simulink model "cddDcmApp"  (R2022b)
settings: Solver=FixedStepDiscrete  StartTime=0.0  StopTime=10.0  FixedStep=0.02  RelTol=1e-3
undefined symbols (not defined in the file or in its folder): CCPFaultType, DcChlType, HFAChlType, IcuChannelType, MtrRoutStaEnum, P_SignalPartType, P_Std_ReturnType, PowerChlType, SrsAdChlType, VGAChlType
(part 1 of 13)
settings Model: Creator=blue.ge  LastModifiedBy=blue.ge
settings DataIOCC: SaveFormat=StructureWithTime
settings OptimizationCC: DefaultParameterBehavior=Inlined  EnhancedBackFolding=on  PassReuseOutputArgsAs=Individual arguments  ZeroExternalMemoryAtStartup=off  ZeroInternalMemoryAtStartup=off  OptimizeBlockOrder=speed  DifferentSizesBufferReuse=on  OptimizationCustomize=off  EfficientTunableParamExpr=on
settings DebuggingCC: ParameterTunabilityLossMsg=error
settings ModelReferenceCC: ModelReferenceNumInstancesAllowed=Single
settings SFSimCC: MATLABDynamicMemAlloc=off
settings RTWCC: Description=AUTOSAR  SystemTargetFile=autosar.tlc  GenCodeOnly=on  TemplateMakefile=ert_default_tmf
settings CodeAppCC: ForceParamTrailComments=on  IgnoreCustomStorageClasses=off  OperatorAnnotations=on  SimulinkDataObjDesc=on  SFDataObjDesc=on  InsertBlockDesc=on
settings STFCustomTargetCC: TargetLangStandard=C99 (ISO)  MultiwordLength=256  ParMdlRefBuildCompliant=on  CompOptLevelCompliant=on  IncludeMdlTerminateFcn=off  CombineOutputUpdateFcns=on  SuppressErrorStatus=on  ERTCustomFileBanners=on  SupportNonFinite=off  SupportComplex=off  SupportContinuousTime=off  SupportNonInlinedSFcns=off  RemoveResetFunc=on  MATLABClassNameForMDSCustomization=Simulink.SoftwareTarget.ERTCustomization  AutosarCompliant=on  UseToolchainInfoCompliant=on  LookupTableObjectStructAxisOrder=2,1,3,4,...  LUTObjectStructOrderEvenSpacing=Size,Table,Breakpoints  SystemTargetFile=autosar.tlc
settings ERTTargetCC: TargetLangStandard=C99 (ISO)  ConcurrentExecutionCompliant=off  IncludeMdlTerminateFcn=off  SuppressErrorStatus=on  SupportNonFinite=off  SupportComplex=off  ModelStepFunctionPrototypeControlCompliant=off  CPPClassGenCompliant=off  AutosarCompliant=on  LookupTableObjectStructAxisOrder=2,1,3,4,...  LUTObjectStructOrderEvenSpacing=Size,Table,Breakpoints  InlinedParameterPlacement=NonHierarchical  ERTCustomFileTemplate=example_file_process.tlc

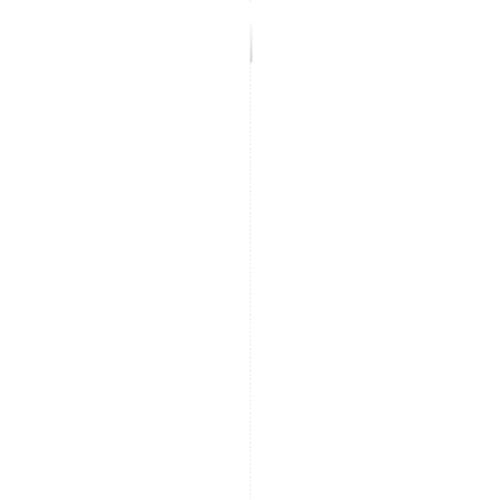
[thumbnail: Simulink's stored preview of the top-level diagram]
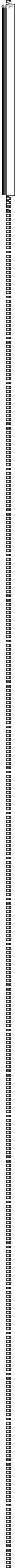
== system cddDcmApp ==
  cddDcmApp_20ms_Runnable : Inport  SampleTime=0.02  OutputFunctionCall=on
  cddDcmApp_Init : Inport  Port=2  OutputFunctionCall=on
  Dcm_Reset_Hard : Inport  Port=3  OutputFunctionCall=on
  Dcm_Reset_JumpToBootLoader : Inport  Port=4  OutputFunctionCall=on
  DiagSessionCtrl_Default : Inport  Port=5  OutputFunctionCall=on
  AACAirClngErrStsNgcc_AACAirClngErrStsNgcc : Inport  Port=6  OutDataTypeStr=Enum: AACAirClngErrSts  PortDimensions=1
  AACAirClngStsNgcc_AACAirClngStsNgcc : Inport  Port=7  OutDataTypeStr=Enum: AACAirClngSts  PortDimensions=1
  AfuFrameSts_AfuFrameSts : Inport  Port=8  OutDataTypeStr=UInt8  PortDimensions=1
  AFUGenErr_AFUGenErr : Inport  Port=9  OutDataTypeStr=Enum: OnOff1  PortDimensions=1
  AirClngErrSts_AirClngErrSts : Inport  Port=10  OutDataTypeStr=Enum: AirClngErrSts  PortDimensions=1
  AirClngSts_AirClngSts : Inport  Port=11  OutDataTypeStr=Enum: AirIonSts  PortDimensions=1
  AirFragFanErr_AirFragFanErr : Inport  Port=12  OutDataTypeStr=Enum: Err1  PortDimensions=1
  AirFragMot1Err_AirFragMot1Err : Inport  Port=13  OutDataTypeStr=Enum: Err1  PortDimensions=1
  AirFragMot2Err_AirFragMot2Err : Inport  Port=14  OutDataTypeStr=Enum: Err1  PortDimensions=1
  AirFragMot3Err_AirFragMot3Err : Inport  Port=15  OutDataTypeStr=Enum: Err1  PortDimensions=1
  AirFragMot4Err_AirFragMot4Err : Inport  Port=16  OutDataTypeStr=Enum: Err1  PortDimensions=1
  AirFragMot5Err_AirFragMot5Err : Inport  Port=17  OutDataTypeStr=Enum: Err1  PortDimensions=1
  AirFragOvrVoltErr_AirFragOvrVoltErr : Inport  Port=18  OutDataTypeStr=Enum: Err1  PortDimensions=1
  AirFragTempErr_AirFragTempErr : Inport  Port=19  OutDataTypeStr=Enum: TempErr  PortDimensions=1
  AirFragUndVoltErr_AirFragUndVoltErr : Inport  Port=20  OutDataTypeStr=Enum: Err1  PortDimensions=1
  AirTFromHeatgEstimdFirstLeForCCM_AirTFromHeatgEstimdFirstLeForCCM : Inport  Port=21  OutDataTypeStr=T30  PortDimensions=1
  AirTFromHeatgEstimdFirstRiForCCM_AirTFromHeatgEstimdFirstRiForCCM : Inport  Port=22  OutDataTypeStr=T30  PortDimensions=1
  AirTFromHeatgEstimdForCCMForRead_AirTFromHeatgEstimdForCCMForRead : Inport  Port=23  OutDataTypeStr=T30  PortDimensions=1
  AirTFromHeatgEstimdReForCCM_AirTFromHeatgEstimdReForCCM : Inport  Port=24  OutDataTypeStr=T30  PortDimensions=1
  AirTFromHeatgEstimdSecLeForCCM_AirTFromHeatgEstimdSecLeForCCM : Inport  Port=25  OutDataTypeStr=T30  PortDimensions=1
  AiuFrameSts_AiuFrameSts : Inport  Port=26  OutDataTypeStr=UInt8  PortDimensions=1
  AmbTFildForClimaNgcc_AmbTFildForClima : Inport  Port=27  OutDataTypeStr=T30  PortDimensions=1
  AmbTRaw_AmbTRaw : Inport  Port=28  OutDataTypeStr=Bus: AmbTWithQly  PortDimensions=1  BusOutputAsStruct=on
  AQSAirQuality_AQSAirQuality : Inport  Port=29  OutDataTypeStr=Enum: AQSAirQuality  PortDimensions=1
  AQSCOxstatus_AQSCOxstatus : Inport  Port=30  OutDataTypeStr=Enum: AQSAirQuality  PortDimensions=1
  AqsFrameSts_AqsFrameSts : Inport  Port=31  OutDataTypeStr=UInt8  PortDimensions=1
  AQSNH3status_AQSNH3status : Inport  Port=32  OutDataTypeStr=Enum: AQSAirQuality  PortDimensions=1
  AQSNoxStatus_AQSNoxStatus : Inport  Port=33  OutDataTypeStr=Enum: AQSAirQuality  PortDimensions=1
  BkpOfDstTrvld_BkpOfDstTrvld : Inport  Port=34  OutDataTypeStr=Len31  PortDimensions=1
  BkpOfDstTrvld_BkpOfDstTrvld_IsUpdated : Inport  Port=35  OutDataTypeStr=boolean  PortDimensions=1
  CarCfgForClimaCtrl_CarCfgForAirClng : Inport  Port=36  OutDataTypeStr=Enum: CarCfgForAirClng  PortDimensions=1
  CarCfgForClimaCtrl_CarCfgForAirClnrAdv : Inport  Port=37  OutDataTypeStr=UInt8  PortDimensions=1
  CarCfgForClimaCtrl_CarCfgForAirFrag : Inport  Port=38  OutDataTypeStr=Enum: CarCfgForAirFrag  PortDimensions=1
  CarCfgForClimaCtrl_CarCfgForAirMtrlSnsr : Inport  Port=39  OutDataTypeStr=Enum: CarCfgForAirMtrlSnsrGly  PortDimensions=1
  CarCfgForClimaCtrl_CarCfgForAirQly : Inport  Port=40  OutDataTypeStr=Enum: CarCfgForAirQlyGly  PortDimensions=1
  CarCfgForClimaCtrl_CarCfgForAmntOfSeat : Inport  Port=41  OutDataTypeStr=Enum: CarCfgForAmntOfSeatTyp3  PortDimensions=1
  CarCfgForClimaCtrl_CarCfgForAutDemst : Inport  Port=42  OutDataTypeStr=UInt8  PortDimensions=1
  CarCfgForClimaCtrl_CarCfgForAuxWtrPmp : Inport  Port=43  OutDataTypeStr=Enum: CarCfgForAuxWtrPmp  PortDimensions=1
  CarCfgForClimaCtrl_CarCfgForBRAND : Inport  Port=44  OutDataTypeStr=UInt8  PortDimensions=1
  CarCfgForClimaCtrl_CarCfgForBypVlvInHvac : Inport  Port=45  OutDataTypeStr=Enum: CarCfgForBypVlvInHvac2  PortDimensions=1
  CarCfgForClimaCtrl_CarCfgForCabinOxgenSupply : Inport  Port=46  OutDataTypeStr=UInt8  PortDimensions=1
  CarCfgForClimaCtrl_CarCfgForClsVlvInHvac : Inport  Port=47  OutDataTypeStr=Enum: CarCfgForClsVlvInHvac  PortDimensions=1
  CarCfgForClimaCtrl_CarCfgForCmprAcTyp : Inport  Port=48  OutDataTypeStr=UInt8  PortDimensions=1
  CarCfgForClimaCtrl_CarCfgForCmptmtClng : Inport  Port=49  OutDataTypeStr=Enum: CarCfgForCmptmtClng  PortDimensions=1
  CarCfgForClimaCtrl_CarCfgForElectricLiftingModule : Inport  Port=50  OutDataTypeStr=UInt8  PortDimensions=1
  CarCfgForClimaCtrl_CarCfgForElecVentAdjmt : Inport  Port=51  OutDataTypeStr=Enum: CarCfgForElecVentAdjmt  PortDimensions=1
  CarCfgForClimaCtrl_CarCfgForExtVentnClimaCtrl : Inport  Port=52  OutDataTypeStr=Enum: CarCfgForExtVentnClimaCtrl  PortDimensions=1
  CarCfgForClimaCtrl_CarCfgForFlcTyp : Inport  Port=53  OutDataTypeStr=Enum: CarCfgForFlcTyp  PortDimensions=1
  CarCfgForClimaCtrl_CarCfgForFloatingDriving : Inport  Port=54  OutDataTypeStr=UInt8  PortDimensions=1
  CarCfgForClimaCtrl_CarCfgForFoldCSD : Inport  Port=55  OutDataTypeStr=Enum: CarCfgForFoldCSD  PortDimensions=1
  CarCfgForClimaCtrl_CarCfgForFuTyp : Inport  Port=56  OutDataTypeStr=Enum: CarCfgForFuTyp  PortDimensions=1
  CarCfgForClimaCtrl_CarCfgForGlassTyp : Inport  Port=57  OutDataTypeStr=Enum: CarCfgForGlassTyp  PortDimensions=1
  CarCfgForClimaCtrl_CarCfgForHeadRadar : Inport  Port=58  OutDataTypeStr=Enum: HeadRadar  PortDimensions=1
  CarCfgForClimaCtrl_CarCfgForHeatPmp : Inport  Port=59  OutDataTypeStr=Enum: CarCfgForHeatPmpTyp  PortDimensions=1
  CarCfgForClimaCtrl_CarCfgForHeatrDurgDrvg : Inport  Port=60  OutDataTypeStr=Enum: CarCfgForHeatrDurgDrvg  PortDimensions=1
  CarCfgForClimaCtrl_CarCfgForHeatrTyp : Inport  Port=61  OutDataTypeStr=UInt8  PortDimensions=1
  CarCfgForClimaCtrl_CarCfgForHvacFilTyp : Inport  Port=62  OutDataTypeStr=Enum: CarCfgForHvacFilTypGly  PortDimensions=1
  CarCfgForClimaCtrl_CarCfgForHvacFlrSnsrForT : Inport  Port=63  OutDataTypeStr=Enum: CarCfgForHvacFlrSnsrForT  PortDimensions=1
  CarCfgForClimaCtrl_CarCfgForHvacHeatrSnsrT : Inport  Port=64  OutDataTypeStr=Enum: CarCfgForHvacHeatrSnsrT  PortDimensions=1
  CarCfgForClimaCtrl_CarCfgForHvacRe : Inport  Port=65  OutDataTypeStr=UInt8  PortDimensions=1
  CarCfgForClimaCtrl_CarCfgForHvacTyp : Inport  Port=66  OutDataTypeStr=UInt8  PortDimensions=1
  CarCfgForClimaCtrl_CarCfgForInteriorViewCamera : Inport  Port=67  OutDataTypeStr=Enum: CarCfgForInteriorViewCamera  PortDimensions=1
  CarCfgForClimaCtrl_CarCfgForLifeDetection : Inport  Port=68  OutDataTypeStr=Enum: LifeDetection  PortDimensions=1
  CarCfgForClimaCtrl_CarCfgForLoVoltElecCfg : Inport  Port=69  OutDataTypeStr=Enum: CarCfgForLoVoltElecCfg  PortDimensions=1
  CarCfgForClimaCtrl_CarCfgForNavSysandIntnmap : Inport  Port=70  OutDataTypeStr=Enum: CarCfgForNavSysandIntnmap  PortDimensions=1
  CarCfgForClimaCtrl_CarCfgForNrOfDoor : Inport  Port=71  OutDataTypeStr=Enum: CarCfgForNrOfDoor2  PortDimensions=1
  CarCfgForClimaCtrl_CarCfgForPipeInFuTank : Inport  Port=72  OutDataTypeStr=Enum: CarCfgForPipeInFuTank  PortDimensions=1
  CarCfgForClimaCtrl_CarCfgForPowerSeats : Inport  Port=73  OutDataTypeStr=Enum: CarCfgForPowerSeats  PortDimensions=1
  CarCfgForClimaCtrl_CarCfgForPreClng : Inport  Port=74  OutDataTypeStr=Enum: CarCfgForPreClng  PortDimensions=1
  CarCfgForClimaCtrl_CarCfgForPrkgCDSNotAvl : Inport  Port=75  OutDataTypeStr=Enum: CarCfgForPrkgCDSNotAvl  PortDimensions=1
  CarCfgForClimaCtrl_CarCfgForPrkgClima : Inport  Port=76  OutDataTypeStr=Enum: CarCfgForPrkgClima  PortDimensions=1
  CarCfgForClimaCtrl_CarCfgForPrpsnTyp : Inport  Port=77  OutDataTypeStr=Enum: CarCfgForPrpsnTypGly  PortDimensions=1
  CarCfgForClimaCtrl_CarCfgForPTCInFrontHVAC : Inport  Port=78  OutDataTypeStr=UInt8  PortDimensions=1
  CarCfgForClimaCtrl_CarCfgForRemEngStrtPreCdn : Inport  Port=79  OutDataTypeStr=Enum: CarCfgForRemEngStrtPreCdn  PortDimensions=1
  CarCfgForClimaCtrl_CarCfgForRoofHatch : Inport  Port=80  OutDataTypeStr=Enum: CarCfgForRoofHatch  PortDimensions=1
  CarCfgForClimaCtrl_CarCfgForSeatHeatgCal : Inport  Port=81  OutDataTypeStr=Enum: CarCfgForSeatHeatgCal1  PortDimensions=1
  CarCfgForClimaCtrl_CarCfgForSeatHeatgForRowFirst : Inport  Port=82  OutDataTypeStr=Enum: CarCfgForSeatHeatgForRowFirst  PortDimensions=1
  CarCfgForClimaCtrl_CarCfgForSeatHeatgForRowSec : Inport  Port=83  OutDataTypeStr=Enum: CarCfgForSeatHeatgForRowSec  PortDimensions=1
  CarCfgForClimaCtrl_CarCfgForSeatVentnForRowFirst : Inport  Port=84  OutDataTypeStr=Enum: CarCfgForSeatVentnForRowFirst1  PortDimensions=1
  CarCfgForClimaCtrl_CarCfgForSeatVentnForRowSec : Inport  Port=85  OutDataTypeStr=Enum: CarCfgForSeatVentnForRowSecGly  PortDimensions=1
  CarCfgForClimaCtrl_CarCfgForSecRowVentnCenter : Inport  Port=86  OutDataTypeStr=Enum: CarCfgForSecRowVentnCenter  PortDimensions=1
  CarCfgForClimaCtrl_CarCfgForSecRowVentnSide : Inport  Port=87  OutDataTypeStr=Enum: CarCfgForSecRowVentnSide  PortDimensions=1
  CarCfgForClimaCtrl_CarCfgForSmallFlowCyc : Inport  Port=88  OutDataTypeStr=Enum: CarCfgForSmallFlowCyc  PortDimensions=1
  CarCfgForClimaCtrl_CarCfgForSmkSnsr : Inport  Port=89  OutDataTypeStr=Enum: CarCfgForSmkSnsr  PortDimensions=1
  CarCfgForClimaCtrl_CarCfgForSnsrForCmptmtT : Inport  Port=90  OutDataTypeStr=Enum: CarCfgForSnsrForCmptmtT  PortDimensions=1
  CarCfgForClimaCtrl_CarCfgForSnsrHumPrsnt : Inport  Port=91  OutDataTypeStr=Enum: CarCfgForSnsrHumPrsnt  PortDimensions=1
  CarCfgForClimaCtrl_CarCfgForStartDevice : Inport  Port=92  OutDataTypeStr=Enum: StartDevice  PortDimensions=1
  CarCfgForClimaCtrl_CarCfgForSteerWhlHeatg : Inport  Port=93  OutDataTypeStr=UInt8  PortDimensions=1
  CarCfgForClimaCtrl_CarCfgForSteerWhlPosn : Inport  Port=94  OutDataTypeStr=Enum: CarCfgForSteerWhlPosn  PortDimensions=1
  CarCfgForClimaCtrl_CarCfgForSunSnsr : Inport  Port=95  OutDataTypeStr=Enum: CarCfgForSunSnsr  PortDimensions=1
  CarCfgForClimaCtrl_CarCfgForTelmNodTyp : Inport  Port=96  OutDataTypeStr=Enum: CarCfgForTelmNodTyp  PortDimensions=1
  CarCfgForClimaCtrl_CarCfgForTEMPEREDSTORAGEBOX : Inport  Port=97  OutDataTypeStr=UInt8  PortDimensions=1
  CarCfgForClimaCtrl_CarCfgForThermMngt : Inport  Port=98  OutDataTypeStr=Enum: CarCfgForThermMngt  PortDimensions=1
  CarCfgForClimaCtrl_CarCfgForVehTyp : Inport  Port=99  OutDataTypeStr=Enum: CarCfgForVehTyp3  PortDimensions=1
  CarCfgForClimaCtrl_CarCfgForVentLiftMotorExiSts : Inport  Port=100  OutDataTypeStr=UInt32  PortDimensions=1
  CarCfgForClimaCtrl_CarCfgForVgaMotorExistSts : Inport  Port=101  OutDataTypeStr=CarCfgForVgaMotorExistSts  PortDimensions=1
  CarCfgForClimaCtrl_CarCfgForWadingAssistSystem : Inport  Port=102  OutDataTypeStr=UInt8  PortDimensions=1
  CarCfgForClimaCtrl_CarCfgForWindHeatd : Inport  Port=103  OutDataTypeStr=Enum: CarCfgForWindHeatd  PortDimensions=1
  CarCfgForClimaCtrl_CarCfgWindFollowHumanMov : Inport  Port=104  OutDataTypeStr=UInt8  PortDimensions=1
  CarTiGlb_CarTiGlb : Inport  Port=105  OutDataTypeStr=CarTiGlb  PortDimensions=1
  CarTiGlb_CarTiGlb_IsUpdated : Inport  Port=106  OutDataTypeStr=boolean  PortDimensions=1
  CcmIntCo2Lvl_CcmIntCo2Lvl : Inport  Port=107  OutDataTypeStr=Enum: Co2Lvl  PortDimensions=1
  CCMLIN1frameSts_CCMLIN1frameSts : Inport  Port=108  OutDataTypeStr=UInt8  PortDimensions=8
  CCMLIN2frameSts_CCMLIN2frameSts : Inport  Port=109  OutDataTypeStr=UInt8  PortDimensions=8
  CCMLIN3frameSts_CCMLIN3frameSts : Inport  Port=110  OutDataTypeStr=UInt8  PortDimensions=8
  CdsFrameSts_CdsFrameSts : Inport  Port=111  OutDataTypeStr=UInt8  PortDimensions=1
  ClimaObsActv_ClimaObsActv : Inport  Port=112  OutDataTypeStr=Boolean  PortDimensions=1
  CmpmtInAirPmAQI_CmpmtInAirPmAQI : Inport  Port=113  OutDataTypeStr=Enum: CmpmtAirPmLvl  PortDimensions=1
  CmpmtInAirPmDnsty_CmpmtInAirPmDnsty : Inport  Port=114  OutDataTypeStr=UInt10  PortDimensions=1
  CmptmtAirTEstimdAtRowFirst_CmptmtAirTEstimdAtRowFirst : Inport  Port=115  OutDataTypeStr=T30  PortDimensions=1
  CmptmtAirTEstimdAtRowFirstIni_CmptmtAirTEstimdAtRowFirstIni : Inport  Port=116  OutDataTypeStr=SInt8  PortDimensions=1
  CmptmtAirTEstimdAtRowSec_CmptmtAirTEstimdAtRowSec : Inport  Port=117  OutDataTypeStr=T30  PortDimensions=1
  CmptmtAirTEstimdAtRowSecIni_CmptmtAirTEstimdAtRowSecIni : Inport  Port=118  OutDataTypeStr=SInt8  PortDimensions=1
  CmptmtAirTEstimdFirstNvRamIni_CmptmtAirTEstimdFirstNvRamIni : Inport  Port=119  OutDataTypeStr=SInt8  PortDimensions=1
  CmptmtAirTEstimdSecNvRamIni_CmptmtAirTEstimdSecNvRamIni : Inport  Port=120  OutDataTypeStr=SInt8  PortDimensions=1
  CmptmtFrntWindDewT_CmptmtFrntWindDewT : Inport  Port=121  OutDataTypeStr=TByCmptmtSnsrRelHum  PortDimensions=1
  CmptmtFrntWindT_CmptmtFrntWindT : Inport  Port=122  OutDataTypeStr=TByCmptmtSnsrRelHum  PortDimensions=1
  CmptmtOxygenConcVluNgcc_CmptmtOxygenConcVluNgcc : Inport  Port=123  OutMin=0  OutMax=100  OutDataTypeStr=Perc9  PortDimensions=1
  CmptmtRelHum_CmptmtRelHum : Inport  Port=124  OutMin=0  OutMax=100  OutDataTypeStr=Perc2  PortDimensions=1
  CmptmtRelHum_CmptmtRelHum_IsUpdated : Inport  Port=125  OutDataTypeStr=boolean  PortDimensions=1
  CmptmtTRearRaw_CmptmtTRearRaw : Inport  Port=126  OutDataTypeStr=Bus: CmptmtTFrnt  PortDimensions=1  BusOutputAsStruct=on
  CoupldCtrlErrAtRowFirstLe_CoupldCtrlErrAtRowFirstLe : Inport  Port=127  OutMin=-256  OutMax=255.9  OutDataTypeStr=T2  PortDimensions=1
  CoupldCtrlErrAtRowFirstRi_CoupldCtrlErrAtRowFirstRi : Inport  Port=128  OutMin=-256  OutMax=255.9  OutDataTypeStr=T2  PortDimensions=1
  CoupldCtrlErrAtRowSecLe_CoupldCtrlErrAtRowSecLe : Inport  Port=129  OutMin=-256  OutMax=255.9  OutDataTypeStr=T2  PortDimensions=1
  CoupldCtrlErrAtRowSecRi_CoupldCtrlErrAtRowSecRi : Inport  Port=130  OutMin=-256  OutMax=255.9  OutDataTypeStr=T2  PortDimensions=1
  DcMotorCurPosVol_DcMotorCurPosVol : Inport  Port=131  OutDataTypeStr=UInt16  PortDimensions=7
  EngT_Ngcc_EngT : Inport  Port=132  OutDataTypeStr=Bus: EngT  PortDimensions=1  BusOutputAsStruct=on
  EvaprTFrntFildForClima_EvaprTFrntFildForClima : Inport  Port=133  OutDataTypeStr=T30  PortDimensions=1
  EvaprTReFildForClima_EvaprTReFildForClima : Inport  Port=134  OutDataTypeStr=T30  PortDimensions=1
  HmiHvacFanLvlFrnt_HmiHvacFanLvlFrnt : Inport  Port=135  OutDataTypeStr=Enum: HmiHvacFanLvl  PortDimensions=1
  HmiHvacFanLvlRe_HmiHvacFanLvlRe : Inport  Port=136  OutDataTypeStr=Enum: HmiHvacFanLvl  PortDimensions=1
  HvacAirTEstimdAtRowFirstLe_HvacAirTEstimdAtRowFirstLe : Inport  Port=137  OutDataTypeStr=T30  PortDimensions=1
  HvacAirTEstimdAtRowFirstRi_HvacAirTEstimdAtRowFirstRi : Inport  Port=138  OutDataTypeStr=T30  PortDimensions=1
  HvacAirTEstimdAtRowSecLe_HvacAirTEstimdAtRowSecLe : Inport  Port=139  OutDataTypeStr=T30  PortDimensions=1
  HvacAirTEstimdAtRowSecRi_HvacAirTEstimdAtRowSecRi : Inport  Port=140  OutDataTypeStr=T30  PortDimensions=1
  HvacAirTReqAtRowFirstLe_HvacAirTReqAtRowFirstLe : Inport  Port=141  OutDataTypeStr=T30  PortDimensions=1
  HvacAirTReqAtRowFirstRi_HvacAirTReqAtRowFirstRi : Inport  Port=142  OutDataTypeStr=T30  PortDimensions=1
  HvacAirTReqAtRowSecLe_HvacAirTReqAtRowSecLe : Inport  Port=143  OutDataTypeStr=T30  PortDimensions=1
  HvacAirTReqAtRowSecRi_HvacAirTReqAtRowSecRi : Inport  Port=144  OutDataTypeStr=T30  PortDimensions=1
  HvacCfgForClimaCtrl_HvacCfgForAQSTypes : Inport  Port=145  OutDataTypeStr=UInt8  PortDimensions=1
  HvacCfgForClimaCtrl_HvacCfgForHvacFrontBlowerType : Inport  Port=146  OutDataTypeStr=HvacCfgForHvacFrontBlowerType  PortDimensions=1
  HvacCfgForClimaCtrl_HvacCfgForHvacLowSideRETXVESts : Inport  Port=147  OutDataTypeStr=UInt8  PortDimensions=1
  HvacCfgForClimaCtrl_HvacCfgForHvacMiscExistSts : Inport  Port=148  OutDataTypeStr=HvacCfgForHvacMiscExistSts  PortDimensions=1
  HvacCfgForClimaCtrl_HvacCfgForHvacMotorExistSts : Inport  Port=149  OutDataTypeStr=HvacCfgForHvacMotorExistSts  PortDimensions=1
  HvacCfgForClimaCtrl_HvacCfgForHvacReBlowerType : Inport  Port=150  OutDataTypeStr=UInt8  PortDimensions=1
  HvacCfgForClimaCtrl_HvacCfgForHvacReVentPstn : Inport  Port=151  OutDataTypeStr=UInt8  PortDimensions=1
  HvacCfgForClimaCtrl_HvacCfgForHvacSensorExistSts : Inport  Port=152  OutDataTypeStr=HvacCfgForHvacSensorExistSts  PortDimensions=1
  HvacCfgForClimaCtrl_HvacCfgForHvacSnsrRCmptmtESts : Inport  Port=153  OutDataTypeStr=UInt8  PortDimensions=1
  HvacCfgForClimaCtrl_HvacCfgForHvacSnsrReHexESts : Inport  Port=154  OutDataTypeStr=UInt8  PortDimensions=1
  HvacFanSet3HvacFanSpd1_HvacFanSet3HvacFanSpd1 : Inport  Port=155  OutDataTypeStr=BlowerSpd  PortDimensions=1
  HvacFanSts2HvacFanBattI2_HvacFanSts2HvacFanBattI2 : Inport  Port=156  OutMin=0  OutMax=63.25  OutDataTypeStr=BlowerReI  PortDimensions=1
  HvacFanSts2HvacFanBattU2_HvacFanSts2HvacFanBattU2 : Inport  Port=157  OutMin=0  OutMax=25.400000000000002  OutDataTypeStr=U8  PortDimensions=1
  HvacFanSts2HvacFanMotU2_HvacFanSts2HvacFanMotU2 : Inport  Port=158  OutMin=0  OutMax=25.400000000000002  OutDataTypeStr=U8  PortDimensions=1
  HvacFanSts2HvacFanReSts1_HvacFanSts2HvacFanReSts1 : Inport  Port=159  OutDataTypeStr=Enum: BlowerReErrSts  PortDimensions=1
  HvacFanSts2HvacFanReSts2_HvacFanSts2HvacFanReSts2 : Inport  Port=160  OutDataTypeStr=Enum: BlowerReSts  PortDimensions=1
  HvacFanSts3HvacFanBattI_HvacFanSts3HvacFanBattI : Inport  Port=161  OutDataTypeStr=BlowerBattI  PortDimensions=1
  HvacFanSts3HvacFanBattU_HvacFanSts3HvacFanBattU : Inport  Port=162  OutDataTypeStr=U10  PortDimensions=1
  HvacFanSts3HvacFanCircShoOrMotBlkd1_HvacFanSts3HvacFanCircShoOrMotBlkd1 : Inport  Port=163  OutDataTypeStr=HvacFanCircShoOrMotBlkdTyp  PortDimensions=1
  HvacFanSts3HvacFanDiagFlag1_HvacFanSts3HvacFanDiagFlag1 : Inport  Port=164  OutDataTypeStr=Enum: HvacFanDiagFlag  PortDimensions=1
  HvacFanSts3HvacFanILimn_HvacFanSts3HvacFanILimn : Inport  Port=165  OutDataTypeStr=Boolean  PortDimensions=1
  HvacFanSts3HvacFanIntErr1_HvacFanSts3HvacFanIntErr1 : Inport  Port=166  OutDataTypeStr=HvacFanIntErrTyp  PortDimensions=1
  HvacFanSts3HvacFanSpdFd_HvacFanSts3HvacFanSpdFd : Inport  Port=167  OutDataTypeStr=BlowerSpd  PortDimensions=1
  HvacFanSts3HvacFanTErr_HvacFanSts3HvacFanTErr : Inport  Port=168  OutDataTypeStr=Boolean  PortDimensions=1
  HvacFanSts3HvDetd_HvacFanSts3HvDetd : Inport  Port=169  OutDataTypeStr=Boolean  PortDimensions=1
  HvacFanSts3OverCurrErr_HvacFanSts3OverCurrErr : Inport  Port=170  OutDataTypeStr=Boolean  PortDimensions=1
  HvacFanSts3UDetdLo_HvacFanSts3UDetdLo : Inport  Port=171  OutDataTypeStr=Boolean  PortDimensions=1
  HvacFanVoltReqFrnt_HvacFanVoltReqFrnt : Inport  Port=172  OutMin=0  OutMax=100  OutDataTypeStr=Perc9  PortDimensions=1
  HvacFanVoltReqRe_HvacFanVoltReqRe : Inport  Port=173  OutMin=0  OutMax=100  OutDataTypeStr=Perc9  PortDimensions=1
  HvacLpmUFbRe_HvacLpmUFbRe : Inport  Port=174  OutMin=0  OutMax=100  OutDataTypeStr=Perc9  PortDimensions=1
  HvacPwm2MosVFbFrnt_HvacPwm2MosVFbFrnt : Inport  Port=175  OutMin=0  OutMax=100  OutDataTypeStr=Perc9  PortDimensions=1
  HvacPwmSignErr_HvacPwmSignErr : Inport  Port=176  OutDataTypeStr=UInt8  PortDimensions=1
  InPm25FanErr_InPm25FanErr : Inport  Port=177  OutDataTypeStr=Enum: FanErr  PortDimensions=1
  InPm25SensrIntErr_InPm25SensrIntErr : Inport  Port=178  OutDataTypeStr=Enum: VentnActr01UrgcExist  PortDimensions=1
  InPm25SnrTempErr_InPm25SnrTempErr : Inport  Port=179  OutDataTypeStr=Enum: TempErr  PortDimensions=1
  InPm25SnrVoltgRangErr_InPm25SnrVoltgRangErr : Inport  Port=180  OutDataTypeStr=Enum: PmSnrVoltgRangErr  PortDimensions=1
  InPm25SnsrElecErr_InPm25SnsrElecErr : Inport  Port=181  OutDataTypeStr=Enum: PmSnsrErrSts  PortDimensions=1
  IntCo2Conc_IntCo2Conc : Inport  Port=182  OutDataTypeStr=Co2Conc  PortDimensions=1
  IntCo2Lvl_IntCo2Lvl : Inport  Port=183  OutDataTypeStr=Enum: Co2Lvl  PortDimensions=1
  LdAdVal_CurrAdVal : Inport  Port=184  OutDataTypeStr=Bus: LdAd  PortDimensions=1  BusOutputAsStruct=on
  LdAdVal_ValidAdVal : Inport  Port=185  OutDataTypeStr=Bus: LdAd  PortDimensions=1  BusOutputAsStruct=on
  MemReadForCenVentLiftModSts_MemReadForCenVentLiftModSts : Inport  Port=186  OutDataTypeStr=Bus: MemSaveLiftMod  PortDimensions=1  BusOutputAsStruct=on
  MemReadForClimaEgyMgrForDID_MemReadForClimaEgyMgrForDID : Inport  Port=187  OutDataTypeStr=Bus: MemRecForClimaEgyMgr  PortDimensions=1  BusOutputAsStruct=on
  NvMBlockCCMLoadDataArray_NvMBlockCCMLoadDataArray : Inport  Port=188  OutDataTypeStr=UInt8  PortDimensions=62
  NvMBlockDcMtrCalDataArray_NvMBlockDcMtrCalDataArray : Inport  Port=189  OutDataTypeStr=UInt8  PortDimensions=82
  ObsvrAirTOffsFromWindTIni_ObsvrAirTOffsFromWindTIni : Inport  Port=190  OutDataTypeStr=SInt8  PortDimensions=1
  ObsvrCmptmtTIninActv_ObsvrCmptmtTIninActv : Inport  Port=191  OutDataTypeStr=UInt8  PortDimensions=1
  ObsvrCmptmtTRamRxInt_ObsvrCmptmtTRamRxInt : Inport  Port=192  OutDataTypeStr=Enum: VCCNvRamRxSts  PortDimensions=1
  ObsvrInCarTFrntIni_ObsvrInCarTFrntIni : Inport  Port=193  OutDataTypeStr=SInt8  PortDimensions=1
  ObsvrInCarTRearIni_ObsvrInCarTRearIni : Inport  Port=194  OutDataTypeStr=SInt8  PortDimensions=1
  ObsvrIntrMtrlTIni_ObsvrIntrMtrlTIni : Inport  Port=195  OutDataTypeStr=SInt8  PortDimensions=1
  OGMIntErrNgcc_OGMIntErrNgcc : Inport  Port=196  OutDataTypeStr=Enum: Err1  PortDimensions=1
  OGMOvrVoltRangErrNgcc_OGMOvrVoltRangErrNgcc : Inport  Port=197  OutDataTypeStr=Enum: Err1  PortDimensions=1
  OGMTempErrNgcc_OGMTempErrNgcc : Inport  Port=198  OutDataTypeStr=Enum: TempErr  PortDimensions=1
  OGMUndVoltRangErrNgcc_OGMUndVoltRangErrNgcc : Inport  Port=199  OutDataTypeStr=Enum: Err1  PortDimensions=1
  OutdAirQly_OutdAirQly : Inport  Port=200  OutDataTypeStr=Bus: OutdAirQly  PortDimensions=1  BusOutputAsStruct=on
  OxygenConcSnsrElecErrNgcc_OxygenConcSnsrElecErrNgcc : Inport  Port=201  OutDataTypeStr=Enum: OxygenConcSnsrElecErr  PortDimensions=1
  OxygenConcSnsrIntErrNgcc_OxygenConcSnsrIntErrNgcc : Inport  Port=202  OutDataTypeStr=Enum: Err1  PortDimensions=1
  OxygenConcSnsrOvrVoltRangErrNgcc_OxygenConcSnsrOvrVoltRangErrNgcc : Inport  Port=203  OutDataTypeStr=Enum: Err1  PortDimensions=1
  OxygenConcSnsrTempErrNgcc_OxygenConcSnsrTempErrNgcc : Inport  Port=204  OutDataTypeStr=Enum: TempErr  PortDimensions=1
  OxygenConcSnsrUndVoltRangErrNgcc_OxygenConcSnsrUndVoltRangErrNgcc : Inport  Port=205  OutDataTypeStr=Enum: Err1  PortDimensions=1
  PmsiFrameSts_PmsiFrameSts : Inport  Port=206  OutDataTypeStr=UInt8  PortDimensions=1
  ReEvapVlvSts_ReEvapVlvSts : Inport  Port=207  OutDataTypeStr=Enum: EvaprVlvSts  PortDimensions=1
  ReHvacActrElecErr_ReHvacActrElecErr : Inport  Port=208  OutDataTypeStr=Enum: VentnActr01ElecErr  PortDimensions=1
  ReHvacActrOvrTempErr_ReHvacActrOvrTempErr : Inport  Port=209  OutDataTypeStr=Enum: VentnActr01OvrTempErr  PortDimensions=1
  ReHvacActrVoltErr_ReHvacActrVoltErr : Inport  Port=210  OutDataTypeStr=Enum: VentnActr01VoltErr  PortDimensions=1
  RelHumSnsrRelHum_RelHumSnsrRelHum : Inport  Port=211  OutMin=0  OutMax=100  OutDataTypeStr=Perc2  PortDimensions=1
  RhfaFrameSts_RhfaFrameSts : Inport  Port=212  OutDataTypeStr=UInt8  PortDimensions=1
  SensorAdValue_SensorAdValue : Inport  Port=213  OutDataTypeStr=Bus: SensorAd  PortDimensions=1  BusOutputAsStruct=on
  SrsAdVal_CurrAdVal : Inport  Port=214  OutDataTypeStr=Bus: SensorAd  PortDimensions=1  BusOutputAsStruct=on
  SrsAdVal_ValidAdVal : Inport  Port=215  OutDataTypeStr=Bus: SensorAd  PortDimensions=1  BusOutputAsStruct=on
  SunData_SunData : Inport  Port=216  OutDataTypeStr=Bus: SunData  PortDimensions=1  BusOutputAsStruct=on
  SWVersion_SWVersion : Inport  Port=217  OutDataTypeStr=VCCUInt8  PortDimensions=11
  SysServCarCfgSts_SysServCarID : Inport  Port=218  OutDataTypeStr=Enum: SysServCarID  PortDimensions=1
  SysServCarCfgSts_SysServCCPAQSSts : Inport  Port=219  OutDataTypeStr=UInt8  PortDimensions=1
  SysServCarCfgSts_SysServCCPBGMNum : Inport  Port=220  OutDataTypeStr=UInt8  PortDimensions=1
  SysServCarCfgSts_SysServCCPHEXSensorSts : Inport  Port=221  OutDataTypeStr=UInt8  PortDimensions=1
  SysServCarCfgSts_SysServCCPLin2AFUSts : Inport  Port=222  OutDataTypeStr=Boolean  PortDimensions=1
  SysServCarCfgSts_SysServCCPLin2AIUSts : Inport  Port=223  OutDataTypeStr=UInt8  PortDimensions=1
  SysServCarCfgSts_SysServCCPLin2AQSSts : Inport  Port=224  OutDataTypeStr=Boolean  PortDimensions=1
  SysServCarCfgSts_SysServCCPLin2CDSSts : Inport  Port=225  OutDataTypeStr=Boolean  PortDimensions=1
  SysServCarCfgSts_SysServCCPLin2PMSISts : Inport  Port=226  OutDataTypeStr=Boolean  PortDimensions=1
  SysServCarCfgSts_SysServCCPLin3VGASts : Inport  Port=227  OutDataTypeStr=Boolean  PortDimensions=1
  SysServCarCfgSts_SysServDUAirVentSnrSts : Inport  Port=228  OutDataTypeStr=Boolean  PortDimensions=1
  SysServCarCfgSts_SysServDUBlowerPwmSignSts : Inport  Port=229  OutDataTypeStr=Boolean  PortDimensions=1
  SysServCarCfgSts_SysServDUBlowerPwmSignTypeSts : Inport  Port=230  OutDataTypeStr=UInt8  PortDimensions=1
  SysServCarCfgSts_SysServDUBlowerPwmSts : Inport  Port=231  OutDataTypeStr=Boolean  PortDimensions=1
  SysServCarCfgSts_SysServDUDCDefrostSts : Inport  Port=232  OutDataTypeStr=UInt8  PortDimensions=1
  SysServCarCfgSts_SysServDUDCFLMdeSts : Inport  Port=233  OutDataTypeStr=UInt8  PortDimensions=1
  SysServCarCfgSts_SysServDUDCModeSts : Inport  Port=234  OutDataTypeStr=UInt8  PortDimensions=1
  SysServCarCfgSts_SysServDUDCRecSts : Inport  Port=235  OutDataTypeStr=UInt8  PortDimensions=1
  SysServCarCfgSts_SysServDUDCSModeSts : Inport  Port=236  OutDataTypeStr=UInt8  PortDimensions=1
  SysServCarCfgSts_SysServDUDCSTLSts : Inport  Port=237  OutDataTypeStr=UInt8  PortDimensions=1
  SysServCarCfgSts_SysServDUDCTLSts : Inport  Port=238  OutDataTypeStr=UInt8  PortDimensions=1
  SysServCarCfgSts_SysServDUDCTRSts : Inport  Port=239  OutDataTypeStr=UInt8  PortDimensions=1
  SysServCarCfgSts_SysServDUFLFootSts : Inport  Port=240  OutDataTypeStr=UInt8  PortDimensions=1
  SysServCarCfgSts_SysServDUFLVentSts : Inport  Port=241  OutDataTypeStr=UInt8  PortDimensions=1
  SysServCarCfgSts_SysServDUFRFootSts : Inport  Port=242  OutDataTypeStr=UInt8  PortDimensions=1
  SysServCarCfgSts_SysServDUFRVentSts : Inport  Port=243  OutDataTypeStr=UInt8  PortDimensions=1
  SysServCarCfgSts_SysServDUIncarMotorSts : Inport  Port=244  OutDataTypeStr=Boolean  PortDimensions=1
  SysServCarCfgSts_SysServDULin1HBMFSts : Inport  Port=245  OutDataTypeStr=Boolean  PortDimensions=1
  SysServCarCfgSts_SysServDULin4HFAFSts : Inport  Port=246  OutDataTypeStr=UInt32  PortDimensions=1
  SysServCarCfgSts_SysServDULin4HFAFStsH : Inport  Port=247  OutDataTypeStr=UInt32  PortDimensions=1
  SysServCarCfgSts_SysServDUReBlowerPwmSignSts : Inport  Port=248  OutDataTypeStr=UInt8  PortDimensions=1
  SysServCarCfgSts_SysServDUReBlowerPwmSts : Inport  Port=249  OutDataTypeStr=UInt8  PortDimensions=1
  SysServCarCfgSts_SysServDUSFLFootSts : Inport  Port=250  OutDataTypeStr=UInt8  PortDimensions=1
  SysServCarCfgSts_SysServDUSFLVentSts : Inport  Port=251  OutDataTypeStr=UInt8  PortDimensions=1
  SysServCarCfgSts_SysServDUSFRFootSts : Inport  Port=252  OutDataTypeStr=UInt8  PortDimensions=1
  SysServCarCfgSts_SysServDUSFRVentSts : Inport  Port=253  OutDataTypeStr=UInt8  PortDimensions=1
  SysServCarCfgSts_SysServDUSnsrForFCmptmtTSts : Inport  Port=254  OutDataTypeStr=UInt8  PortDimensions=1
  SysServCarCfgSts_SysServDUSnsrForRair : Inport  Port=255  OutDataTypeStr=UInt8  PortDimensions=1
  SysServCarCfgSts_SysServDUSnsrForRCmptmtTSts : Inport  Port=256  OutDataTypeStr=UInt8  PortDimensions=1
  SysServCarCfgSts_SysServFrntHEXSnsrPosn : Inport  Port=257  OutDataTypeStr=UInt8  PortDimensions=1
  SysServCarCfgSts_SysServLowSideRETXVSts : Inport  Port=258  OutDataTypeStr=UInt8  PortDimensions=1
  SysServCarCfgSts_SysServReHEXSnsrPosn : Inport  Port=259  OutDataTypeStr=UInt8  PortDimensions=1
  SysServCarCfgSts_SysServRHFASts : Inport  Port=260  OutDataTypeStr=UInt8  PortDimensions=1
  SysServCarCfgSts_SysServSnsrForFCmptmtTType : Inport  Port=261  OutDataTypeStr=UInt8  PortDimensions=1
  TestRunCriteriaTRC_TestRunCriteriaTRC : Inport  Port=262  OutDataTypeStr=UInt8  PortDimensions=8
  TiOfDeactvnForClimaObs_TiOfDeactvnForClimaObs : Inport  Port=263  OutDataTypeStr=Ti33  PortDimensions=1
  VbmrFrameSts_VbmrFrameSts : Inport  Port=264  OutDataTypeStr=UInt8  PortDimensions=1
  VehBattU_VehBattU : Inport  Port=265  OutDataTypeStr=Bus: SysU  PortDimensions=1  BusOutputAsStruct=on
  VehBattU_VehBattU_IsUpdated : Inport  Port=266  OutDataTypeStr=boolean  PortDimensions=1
  VehModMngtGlbSafe1_VehModMngtGlbSafe1 : Inport  Port=267  OutDataTypeStr=Bus: VehModMngtGlbSafe1  PortDimensions=1  BusOutputAsStruct=on
  VehModMngtGlbSafe1_VehModMngtGlbSafe1_IsUpdated : Inport  Port=268  OutDataTypeStr=boolean  PortDimensions=1
  VentnActr01ElecErr_VentnActr01ElecErr : Inport  Port=269  OutDataTypeStr=Enum: VentnActr01ElecErr  PortDimensions=1
  VentnActr01OvrTempErr_VentnActr01OvrTempErr : Inport  Port=270  OutDataTypeStr=Enum: VentnActr01OvrTempErr  PortDimensions=1
  VentnActr01VoltErr_VentnActr01VoltErr : Inport  Port=271  OutDataTypeStr=Enum: VentnActr01VoltErr  PortDimensions=1
  VentnActr02ElecErr_VentnActr02ElecErr : Inport  Port=272  OutDataTypeStr=Enum: VentnActr01ElecErr  PortDimensions=1
  VentnActr02OvrTempErr_VentnActr02OvrTempErr : Inport  Port=273  OutDataTypeStr=Enum: VentnActr01OvrTempErr  PortDimensions=1
  VentnActr02VoltErr_VentnActr02VoltErr : Inport  Port=274  OutDataTypeStr=Enum: VentnActr01VoltErr  PortDimensions=1